simulink model "MX_Gateway"  (Simulink 9.0)
settings: Solver=FixedStepDiscrete  StartTime=0.0  StopTime=100  FixedStep=0.01  RelTol=1e-3
values from neighbouring files (symbols this model uses):
  ACTIVE = Bobcat.Parameter; CreateDefine( ACTIVE                         ,1                          ,'Define' ,'defines.h' )    [defines.m]
  ACTIVE_MODE_ONE = Bobcat.Parameter; CreateDefine( ACTIVE_MODE_ONE                ,19                         ,'Define' ,'defines.h' )    [defines.m]
  AUTHENTICATED = Bobcat.Parameter; CreateDefine( AUTHENTICATED                  ,1                          ,'Define' ,'defines.h' )    [defines.m]
  AUX_EMEA_CONTROLS = Bobcat.Parameter; CreateDefine( AUX_EMEA_CONTROLS  ,1   ,'Define' ,'defines.h' )    [enums.m]
  AUX_FLOW_HIGH = Bobcat.Parameter; CreateDefine( AUX_FLOW_HIGH           ,4  ,'Define' ,'defines.h' )    [enums.m]
  AUX_FLOW_INACTIVE = Bobcat.Parameter; CreateDefine( AUX_FLOW_INACTIVE       ,0  ,'Define' ,'defines.h' )    [enums.m]
  AUX_FLOW_LOW = Bobcat.Parameter; CreateDefine( AUX_FLOW_LOW            ,1  ,'Define' ,'defines.h' )    [enums.m]
  AUX_FLOW_MEDIUM = Bobcat.Parameter; CreateDefine( AUX_FLOW_MEDIUM         ,2  ,'Define' ,'defines.h' )    [enums.m]
  AUX_FLOW_STANDARD = Bobcat.Parameter; CreateDefine( AUX_FLOW_STANDARD       ,3  ,'Define' ,'defines.h' )    [enums.m]
  AUX_NON_FUNCTIONAL = Bobcat.Parameter; CreateDefine( AUX_NON_FUNCTIONAL ,255 ,'Define' ,'defines.h' )    [enums.m]
  BASE_ACTIVE = Bobcat.Parameter; CreateDefine( BASE_ACTIVE                    ,253                        ,'Define' ,'defines.h' )    [defines.m]
  BASE_DETENT = Bobcat.Parameter; CreateDefine( BASE_DETENT                    ,1                          ,'Define' ,'defines.h' )    [defines.m]
  BEEP_NONE = Bobcat.Parameter; CreateDefine( BEEP_NONE                      ,255                        ,'Define' ,'defines.h' )    [defines.m]
  CD_AIR_FILTER_DEBOUNCE_NORMAL = Bobcat.Parameter; CreateAliasCD( CD_AIR_FILTER_DEBOUNCE_NORMAL                        ,uint16(500)                ,'ConstantDataPointer' ,'CONSTANT_DATA' ,'AIR_FILTER_DEBOUNCE_NORMAL')    [cdata.m]    (also in hc12_cdata.m; m4_cdata.m)
  CD_AIR_FILTER_DEBOUNCE_NOT_CONNECTED = Bobcat.Parameter; CreateAliasCD( CD_AIR_FILTER_DEBOUNCE_NOT_CONNECTED                 ,uint16(3000)               ,'ConstantDataPointer' ,'CONSTANT_DATA' ,'AIR_FILTER_DEBOUNCE_NOT_CONNECTED')    [cdata.m]    (also in hc12_cdata.m; m4_cdata.m)
  CD_AIR_FILTER_DEBOUNCE_PLUGGED = Bobcat.Parameter; CreateAliasCD( CD_AIR_FILTER_DEBOUNCE_PLUGGED                       ,uint16(3000)               ,'ConstantDataPointer' ,'CONSTANT_DATA' ,'AIR_FILTER_DEBOUNCE_PLUGGED')    [cdata.m]    (also in hc12_cdata.m; m4_cdata.m)
  CD_AIR_FILTER_FUNCTION = Bobcat.Parameter; CreateAliasCD( CD_AIR_FILTER_FUNCTION                               ,uint8(255)                 ,'ConstantDataPointer' ,'CONSTANT_DATA' ,'AIR_FILTER_FUNCTION')    [cdata.m]    (also in hc12_cdata.m; m4_cdata.m)
  CD_AIR_FILTER_PLUGGED_WEIGHT = Bobcat.Parameter; CreateAliasCD( CD_AIR_FILTER_PLUGGED_WEIGHT                         ,uint8(2)                   ,'ConstantDataPointer' ,'CONSTANT_DATA' ,'AIR_FILTER_PLUGGED_WEIGHT')    [cdata.m]    (also in hc12_cdata.m; m4_cdata.m)
  CD_AIR_FILTER_UNPLUGGED_WEIGHT = Bobcat.Parameter; CreateAliasCD( CD_AIR_FILTER_UNPLUGGED_WEIGHT                       ,uint8(1)                   ,'ConstantDataPointer' ,'CONSTANT_DATA' ,'AIR_FILTER_UNPLUGGED_WEIGHT')    [cdata.m]    (also in hc12_cdata.m; m4_cdata.m)
  CD_AUTOIDLE_ARMBAR_DELAY = Bobcat.Parameter; CreateAliasCD( CD_AUTOIDLE_ARMBAR_DELAY                             ,uint8(2)                   ,'ConstantDataPointer' ,'CONSTANT_DATA' ,'AUTOIDLE_ARMBAR_DELAY')    [cdata.m]    (also in hc12_cdata.m; m4_cdata.m)
  CD_AUTOIDLE_FUNCTION = Bobcat.Parameter; CreateAliasCD( CD_AUTOIDLE_FUNCTION                                 ,uint8(255)                 ,'ConstantDataPointer' ,'CONSTANT_DATA' ,'AUTOIDLE_FUNCTION')    [cdata.m]    (also in hc12_cdata.m; m4_cdata.m)
  CD_AUTOIDLE_IDLE_DELAY = Bobcat.Parameter; CreateAliasCD( CD_AUTOIDLE_IDLE_DELAY                               ,uint8(40)                  ,'ConstantDataPointer' ,'CONSTANT_DATA' ,'AUTOIDLE_IDLE_DELAY')    [cdata.m]    (also in hc12_cdata.m; m4_cdata.m)
  CD_AUTOIDLE_LOW_IDLE_START = Bobcat.Parameter; CreateAliasCD( CD_AUTOIDLE_LOW_IDLE_START                           ,uint8(255)                 ,'ConstantDataPointer' ,'CONSTANT_DATA' ,'AUTOIDLE_LOW_IDLE_START')    [cdata.m]    (also in hc12_cdata.m; m4_cdata.m)
  CD_AUTOIDLE_PRESSURE_OFF = Bobcat.Parameter; CreateAliasCD( CD_AUTOIDLE_PRESSURE_OFF                             ,uint16(300)                ,'ConstantDataPointer' ,'CONSTANT_DATA' ,'AUTOIDLE_PRESSURE_OFF')    [cdata.m]    (also in hc12_cdata.m; m4_cdata.m)
  CD_AUTOIDLE_PRESSURE_ON = Bobcat.Parameter; CreateAliasCD( CD_AUTOIDLE_PRESSURE_ON                              ,uint16(270)                ,'ConstantDataPointer' ,'CONSTANT_DATA' ,'AUTOIDLE_PRESSURE_ON')    [cdata.m]    (also in hc12_cdata.m; m4_cdata.m)
  CD_AUTOSHIFT_FUNCTION = Bobcat.Parameter; CreateAliasCD( CD_AUTOSHIFT_FUNCTION                                ,uint8(255)                 ,'ConstantDataPointer' ,'CONSTANT_DATA' ,'AUTOSHIFT_FUNCTION')    [cdata.m]    (also in hc12_cdata.m; m4_cdata.m)
  CD_AUTOSHIFT_PHIGH1 = Bobcat.Parameter; CreateAliasCD( CD_AUTOSHIFT_PHIGH1                                  ,uint16(205)                ,'ConstantDataPointer' ,'CONSTANT_DATA' ,'AUTOSHIFT_PHIGH1')    [cdata.m]    (also in hc12_cdata.m; m4_cdata.m)
  CD_AUTOSHIFT_PHIGH2 = Bobcat.Parameter; CreateAliasCD( CD_AUTOSHIFT_PHIGH2                                  ,uint16(193)                ,'ConstantDataPointer' ,'CONSTANT_DATA' ,'AUTOSHIFT_PHIGH2')    [cdata.m]    (also in hc12_cdata.m; m4_cdata.m)
  CD_AUTOSHIFT_PHIGH_TEMP1 = Bobcat.Parameter; CreateAliasCD( CD_AUTOSHIFT_PHIGH_TEMP1                             ,int16(70)                  ,'ConstantDataPointer' ,'CONSTANT_DATA' ,'AUTOSHIFT_PHIGH_TEMP1')    [cdata.m]    (also in hc12_cdata.m; m4_cdata.m)
  CD_AUTOSHIFT_PHIGH_TEMP2 = Bobcat.Parameter; CreateAliasCD( CD_AUTOSHIFT_PHIGH_TEMP2                             ,int16(160)                 ,'ConstantDataPointer' ,'CONSTANT_DATA' ,'AUTOSHIFT_PHIGH_TEMP2')    [cdata.m]    (also in hc12_cdata.m; m4_cdata.m)
  CD_AUTOSHIFT_PLOW1 = Bobcat.Parameter; CreateAliasCD( CD_AUTOSHIFT_PLOW1                                   ,uint16(130)                ,'ConstantDataPointer' ,'CONSTANT_DATA' ,'AUTOSHIFT_PLOW1')    [cdata.m]    (also in hc12_cdata.m; m4_cdata.m)
  CD_AUTOSHIFT_PLOW2 = Bobcat.Parameter; CreateAliasCD( CD_AUTOSHIFT_PLOW2                                   ,uint16(100)                ,'ConstantDataPointer' ,'CONSTANT_DATA' ,'AUTOSHIFT_PLOW2')    [cdata.m]    (also in hc12_cdata.m; m4_cdata.m)
  CD_AUTOSHIFT_PLOW_TEMP1 = Bobcat.Parameter; CreateAliasCD( CD_AUTOSHIFT_PLOW_TEMP1                              ,int16(20)                  ,'ConstantDataPointer' ,'CONSTANT_DATA' ,'AUTOSHIFT_PLOW_TEMP1')    [cdata.m]    (also in hc12_cdata.m; m4_cdata.m)
  CD_AUTOSHIFT_PLOW_TEMP2 = Bobcat.Parameter; CreateAliasCD( CD_AUTOSHIFT_PLOW_TEMP2                              ,int16(80)                  ,'ConstantDataPointer' ,'CONSTANT_DATA' ,'AUTOSHIFT_PLOW_TEMP2')    [cdata.m]    (also in hc12_cdata.m; m4_cdata.m)
  CD_AUTOSHIFT_TRAVEL_DELAY = Bobcat.Parameter; CreateAliasCD( CD_AUTOSHIFT_TRAVEL_DELAY                            ,uint8(32)                  ,'ConstantDataPointer' ,'CONSTANT_DATA' ,'AUTOSHIFT_TRAVEL_DELAY')    [cdata.m]    (also in hc12_cdata.m; m4_cdata.m)
  CD_AUX2_CRACKPOINT_CURRENT = Bobcat.Parameter; CreateAliasCD( CD_AUX2_CRACKPOINT_CURRENT                           ,fi(0,0,16,12,'hex','0c28') ,'ConstantDataPointer' ,'CONSTANT_DATA' ,'AUX2_CRACKPOINT_CURRENT')    [cdata.m]    (also in hc12_cdata.m; m4_cdata.m)
  CD_AUX2_FLOW_LOW_FUNCTION = Bobcat.Parameter; CreateAliasCD( CD_AUX2_FLOW_LOW_FUNCTION                            ,uint8(255)                 ,'ConstantDataPointer' ,'CONSTANT_DATA' ,'AUX2_FLOW_LOW_FUNCTION')    [cdata.m]    (also in hc12_cdata.m; m4_cdata.m)
  CD_AUX2_FLOW_MED_FUNCTION = Bobcat.Parameter; CreateAliasCD( CD_AUX2_FLOW_MED_FUNCTION                            ,uint8(255)                 ,'ConstantDataPointer' ,'CONSTANT_DATA' ,'AUX2_FLOW_MED_FUNCTION')    [cdata.m]    (also in hc12_cdata.m; m4_cdata.m)
  CD_AUX2_HI_FLOW_PERCENT = Bobcat.Parameter; CreateAliasCD( CD_AUX2_HI_FLOW_PERCENT                              ,fi(0,0,16,16,'hex','ffff') ,'ConstantDataPointer' ,'CONSTANT_DATA' ,'AUX2_HI_FLOW_PERCENT')    [cdata.m]    (also in hc12_cdata.m; m4_cdata.m)
  CD_AUX2_LOW_FLOW_PERCENT = Bobcat.Parameter; CreateAliasCD( CD_AUX2_LOW_FLOW_PERCENT                             ,fi(0,0,16,16,'hex','7333') ,'ConstantDataPointer' ,'CONSTANT_DATA' ,'AUX2_LOW_FLOW_PERCENT')    [cdata.m]    (also in hc12_cdata.m; m4_cdata.m)
  CD_AUX2_MAX_FLOW_CURRENT = Bobcat.Parameter; CreateAliasCD( CD_AUX2_MAX_FLOW_CURRENT                             ,fi(0,0,16,12,'hex','14cc') ,'ConstantDataPointer' ,'CONSTANT_DATA' ,'AUX2_MAX_FLOW_CURRENT')    [cdata.m]    (also in hc12_cdata.m; m4_cdata.m)
  CD_AUX2_MED_FLOW_PERCENT = Bobcat.Parameter; CreateAliasCD( CD_AUX2_MED_FLOW_PERCENT                             ,fi(0,0,16,16,'hex','9999') ,'ConstantDataPointer' ,'CONSTANT_DATA' ,'AUX2_MED_FLOW_PERCENT')    [cdata.m]    (also in hc12_cdata.m; m4_cdata.m)
  CD_AUX_CRACKPOINT_CURRENT = Bobcat.Parameter; CreateAliasCD( CD_AUX_CRACKPOINT_CURRENT                            ,fi(0,0,16,12,'hex','0000') ,'ConstantDataPointer' ,'CONSTANT_DATA' ,'AUX_CRACKPOINT_CURRENT')    [cdata.m]    (also in hc12_cdata.m; m4_cdata.m)
  CD_AUX_DETENT_FLASH_TIME = Bobcat.Parameter; CreateAliasCD( CD_AUX_DETENT_FLASH_TIME                             ,uint16(72)                 ,'ConstantDataPointer' ,'CONSTANT_DATA' ,'AUX_DETENT_FLASH_TIME')    [cdata.m]    (also in hc12_cdata.m; m4_cdata.m)
  CD_AUX_DETENT_HOLD_TIME = Bobcat.Parameter; CreateAliasCD( CD_AUX_DETENT_HOLD_TIME                              ,uint16(100)                ,'ConstantDataPointer' ,'CONSTANT_DATA' ,'AUX_DETENT_HOLD_TIME')    [cdata.m]    (also in hc12_cdata.m; m4_cdata.m)
  CD_AUX_FULL_ON_AUX1 = Bobcat.Parameter; CreateAliasCD( CD_AUX_FULL_ON_AUX1                                  ,fi(0,0,16,12,'hex','10cd') ,'ConstantDataPointer' ,'CONSTANT_DATA' ,'AUX_FULL_ON_AUX1')    [cdata.m]    (also in hc12_cdata.m; m4_cdata.m)
  CD_AUX_FULL_ON_AUX2 = Bobcat.Parameter; CreateAliasCD( CD_AUX_FULL_ON_AUX2                                  ,fi(0,0,16,12,'hex','139d') ,'ConstantDataPointer' ,'CONSTANT_DATA' ,'AUX_FULL_ON_AUX2')    [cdata.m]    (also in hc12_cdata.m; m4_cdata.m)
  CD_AUX_FULL_ON_AUX3 = Bobcat.Parameter; CreateAliasCD( CD_AUX_FULL_ON_AUX3                                  ,fi(0,0,16,12,'hex','17d7') ,'ConstantDataPointer' ,'CONSTANT_DATA' ,'AUX_FULL_ON_AUX3')    [cdata.m]    (also in hc12_cdata.m; m4_cdata.m)
  CD_AUX_FUNCTION = Bobcat.Parameter; CreateAliasCD( CD_AUX_FUNCTION                                      ,uint8(0)                   ,'ConstantDataPointer' ,'CONSTANT_DATA' ,'AUX_FUNCTION')    [cdata.m]    (also in hc12_cdata.m; m4_cdata.m)
  CD_AUX_RATE_LIMIT_CRACKPOINT = Bobcat.Parameter; CreateAliasCD( CD_AUX_RATE_LIMIT_CRACKPOINT                         ,fi(0,0,16,12,'hex','0800') ,'ConstantDataPointer' ,'CONSTANT_DATA' ,'AUX_RATE_LIMIT_CRACKPOINT')    [cdata.m]    (also in hc12_cdata.m; m4_cdata.m)
  CD_AUX_RATE_LIMIT_CRACKPOINT_RATE = Bobcat.Parameter; CreateAliasCD( CD_AUX_RATE_LIMIT_CRACKPOINT_RATE                    ,fi(0,1,32,12,'int',102400) ,'ConstantDataPointer' ,'CONSTANT_DATA' ,'AUX_RATE_LIMIT_CRACKPOINT_RATE')    [cdata.m]    (also in hc12_cdata.m; m4_cdata.m)
  CD_AUX_RATE_LIMIT_FUNCTION = Bobcat.Parameter; CreateAliasCD( CD_AUX_RATE_LIMIT_FUNCTION                           ,uint8(255)                 ,'ConstantDataPointer' ,'CONSTANT_DATA' ,'AUX_RATE_LIMIT_FUNCTION')    [cdata.m]    (also in hc12_cdata.m; m4_cdata.m)
  CD_AUX_RATE_LIMIT_MAX_ENG_SPD = Bobcat.Parameter; CreateAliasCD( CD_AUX_RATE_LIMIT_MAX_ENG_SPD                        ,uint16(1600)               ,'ConstantDataPointer' ,'CONSTANT_DATA' ,'AUX_RATE_LIMIT_MAX_ENG_SPD')    [cdata.m]    (also in hc12_cdata.m; m4_cdata.m)
  CD_AUX_RATE_LIMIT_MIN_ENG_SPD = Bobcat.Parameter; CreateAliasCD( CD_AUX_RATE_LIMIT_MIN_ENG_SPD                        ,uint16(100)                ,'ConstantDataPointer' ,'CONSTANT_DATA' ,'AUX_RATE_LIMIT_MIN_ENG_SPD')    [cdata.m]    (also in hc12_cdata.m; m4_cdata.m)
  CD_AUX_RATE_LIMIT_NORMAL_RATE = Bobcat.Parameter; CreateAliasCD( CD_AUX_RATE_LIMIT_NORMAL_RATE                        ,fi(0,1,32,12,'int',2048)   ,'ConstantDataPointer' ,'CONSTANT_DATA' ,'AUX_RATE_LIMIT_NORMAL_RATE')    [cdata.m]    (also in hc12_cdata.m; m4_cdata.m)
  CD_AUXPOT_CAL_DEADBAND_HIGH = Bobcat.Parameter; CreateAliasCD( CD_AUXPOT_CAL_DEADBAND_HIGH                          ,uint16(574)                ,'ConstantDataPointer' ,'CONSTANT_DATA' ,'AUXPOT_CAL_DEADBAND_HIGH')    [cdata.m]    (also in hc12_cdata.m; m4_cdata.m)
  CD_AUXPOT_CAL_DEADBAND_LOW = Bobcat.Parameter; CreateAliasCD( CD_AUXPOT_CAL_DEADBAND_LOW                           ,uint16(424)                ,'ConstantDataPointer' ,'CONSTANT_DATA' ,'AUXPOT_CAL_DEADBAND_LOW')    [cdata.m]    (also in hc12_cdata.m; m4_cdata.m)
  CD_AUXPOT_CENTER_RATIO = Bobcat.Parameter; CreateAliasCD( CD_AUXPOT_CENTER_RATIO                               ,uint16(500)                ,'ConstantDataPointer' ,'CONSTANT_DATA' ,'AUXPOT_CENTER_RATIO')    [cdata.m]    (also in hc12_cdata.m; m4_cdata.m)
  CD_AUXPOT_DEADBAND = Bobcat.Parameter; CreateAliasCD( CD_AUXPOT_DEADBAND                                   ,uint8(38)                  ,'ConstantDataPointer' ,'CONSTANT_DATA' ,'AUXPOT_DEADBAND')    [cdata.m]    (also in hc12_cdata.m; m4_cdata.m)
  CD_AUXPOT_FUNCTION = Bobcat.Parameter; CreateAliasCD( CD_AUXPOT_FUNCTION                                   ,uint8(0)                   ,'ConstantDataPointer' ,'CONSTANT_DATA' ,'AUXPOT_FUNCTION')    [cdata.m]    (also in hc12_cdata.m; m4_cdata.m)
  CD_AUXPOT_RATIO_GAIN = Bobcat.Parameter; CreateAliasCD( CD_AUXPOT_RATIO_GAIN                                 ,uint16(1000)               ,'ConstantDataPointer' ,'CONSTANT_DATA' ,'AUXPOT_RATIO_GAIN')    [cdata.m]    (also in hc12_cdata.m; m4_cdata.m)
  CD_CAN_THROTTLE_FUNCTION = Bobcat.Parameter; CreateAliasCD( CD_CAN_THROTTLE_FUNCTION                             ,uint8(255)                 ,'ConstantDataPointer' ,'CONSTANT_DATA' ,'CAN_THROTTLE_FUNCTION')    [cdata.m]    (also in hc12_cdata.m; m4_cdata.m)
  CD_CAN_THROTTLE_REVERSE = Bobcat.Parameter; CreateAliasCD( CD_CAN_THROTTLE_REVERSE                              ,uint8(255)                 ,'ConstantDataPointer' ,'CONSTANT_DATA' ,'CAN_THROTTLE_REVERSE')    [cdata.m]    (also in hc12_cdata.m; m4_cdata.m)
  CD_CONSOLE_DEBOUNCE_ABSENT = Bobcat.Parameter; CreateAliasCD( CD_CONSOLE_DEBOUNCE_ABSENT                           ,uint16(12)                 ,'ConstantDataPointer' ,'CONSTANT_DATA' ,'CONSOLE_DEBOUNCE_ABSENT')    [cdata.m]    (also in hc12_cdata.m; m4_cdata.m)
  CD_CONSOLE_DEBOUNCE_OORH = Bobcat.Parameter; CreateAliasCD( CD_CONSOLE_DEBOUNCE_OORH                             ,uint16(12)                 ,'ConstantDataPointer' ,'CONSTANT_DATA' ,'CONSOLE_DEBOUNCE_OORH')    [cdata.m]    (also in hc12_cdata.m; m4_cdata.m)
  CD_CONSOLE_DEBOUNCE_OORL = Bobcat.Parameter; CreateAliasCD( CD_CONSOLE_DEBOUNCE_OORL                             ,uint16(12)                 ,'ConstantDataPointer' ,'CONSTANT_DATA' ,'CONSOLE_DEBOUNCE_OORL')    [cdata.m]    (also in hc12_cdata.m; m4_cdata.m)
  CD_CONSOLE_DEBOUNCE_PRESENT = Bobcat.Parameter; CreateAliasCD( CD_CONSOLE_DEBOUNCE_PRESENT                          ,uint16(12)                 ,'ConstantDataPointer' ,'CONSTANT_DATA' ,'CONSOLE_DEBOUNCE_PRESENT')    [cdata.m]    (also in hc12_cdata.m; m4_cdata.m)
  CD_CONSOLE_FUNCTION = Bobcat.Parameter; CreateAliasCD( CD_CONSOLE_FUNCTION                                  ,uint8(0)                   ,'ConstantDataPointer' ,'CONSTANT_DATA' ,'CONSOLE_FUNCTION')    [cdata.m]    (also in hc12_cdata.m; m4_cdata.m)
  CD_CONSOLE_OORH = Bobcat.Parameter; CreateAliasCD( CD_CONSOLE_OORH                                      ,uint16(740)                ,'ConstantDataPointer' ,'CONSTANT_DATA' ,'CONSOLE_OORH')    [cdata.m]    (also in hc12_cdata.m; m4_cdata.m)
  CD_CONSOLE_OORL = Bobcat.Parameter; CreateAliasCD( CD_CONSOLE_OORL                                      ,uint16(10)                 ,'ConstantDataPointer' ,'CONSTANT_DATA' ,'CONSOLE_OORL')    [cdata.m]    (also in hc12_cdata.m; m4_cdata.m)
  CD_CONSOLE_PRESENT_HIGH = Bobcat.Parameter; CreateAliasCD( CD_CONSOLE_PRESENT_HIGH                              ,uint16(515)                ,'ConstantDataPointer' ,'CONSTANT_DATA' ,'CONSOLE_PRESENT_HIGH')    [cdata.m]    (also in hc12_cdata.m; m4_cdata.m)
  CD_CONSOLE_PRESENT_LOW = Bobcat.Parameter; CreateAliasCD( CD_CONSOLE_PRESENT_LOW                               ,uint16(375)                ,'ConstantDataPointer' ,'CONSTANT_DATA' ,'CONSOLE_PRESENT_LOW')    [cdata.m]    (also in hc12_cdata.m; m4_cdata.m)
  CD_DELUXE_GEN5_5_FUNCTION = Bobcat.Parameter; CreateAliasCD( CD_DELUXE_GEN5_5_FUNCTION                            ,uint8(255)                 ,'ConstantDataPointer' ,'CONSTANT_DATA' ,'DELUXE_GEN5_5_FUNCTION')    [cdata.m]    (also in hc12_cdata.m; m4_cdata.m)
  CD_DELUXE_GEN5_FUNCTION = Bobcat.Parameter; CreateAliasCD( CD_DELUXE_GEN5_FUNCTION                              ,uint8(255)                 ,'ConstantDataPointer' ,'CONSTANT_DATA' ,'DELUXE_GEN5_FUNCTION')    [cdata.m]    (also in hc12_cdata.m; m4_cdata.m)
  CD_DERATE_ENGINE_SPEED_DEBOUNCE_HIGH = Bobcat.Parameter; CreateAliasCD( CD_DERATE_ENGINE_SPEED_DEBOUNCE_HIGH                 ,uint16(200)                ,'ConstantDataPointer' ,'CONSTANT_DATA' ,'DERATE_ENGINE_SPEED_DEBOUNCE_HIGH')    [cdata.m]    (also in hc12_cdata.m; m4_cdata.m)
  CD_DERATE_ENGINE_SPEED_DEBOUNCE_SHUTDOWN = Bobcat.Parameter; CreateAliasCD( CD_DERATE_ENGINE_SPEED_DEBOUNCE_SHUTDOWN             ,uint16(1000)               ,'ConstantDataPointer' ,'CONSTANT_DATA' ,'DERATE_ENGINE_SPEED_DEBOUNCE_SHUTDOWN')    [cdata.m]    (also in hc12_cdata.m; m4_cdata.m)
  CD_DERATE_ENGINE_SPEED_HIGH_OFFSET = Bobcat.Parameter; CreateAliasCD( CD_DERATE_ENGINE_SPEED_HIGH_OFFSET                   ,uint16(200)                ,'ConstantDataPointer' ,'CONSTANT_DATA' ,'DERATE_ENGINE_SPEED_HIGH_OFFSET')    [cdata.m]    (also in hc12_cdata.m; m4_cdata.m)
  CD_DERATE_METHOD = Bobcat.Parameter; CreateAliasCD( CD_DERATE_METHOD                                     ,uint8(0)                   ,'ConstantDataPointer' ,'CONSTANT_DATA' ,'DERATE_METHOD')    [cdata.m]    (also in hc12_cdata.m; m4_cdata.m)
  CD_DUMP_VALVE_FUNCTION = Bobcat.Parameter; CreateAliasCD( CD_DUMP_VALVE_FUNCTION                               ,uint8(255)                 ,'ConstantDataPointer' ,'CONSTANT_DATA' ,'DUMP_VALVE_FUNCTION')    [cdata.m]    (also in hc12_cdata.m; m4_cdata.m)
  CD_DUMP_VALVE_ON_CURRENT = Bobcat.Parameter; CreateAliasCD( CD_DUMP_VALVE_ON_CURRENT                             ,fi(0,0,16,12,'hex','ffff') ,'ConstantDataPointer' ,'CONSTANT_DATA' ,'DUMP_VALVE_ON_CURRENT')    [cdata.m]    (also in hc12_cdata.m; m4_cdata.m)
  CD_ECO_MODE_THROTTLE_REDUCTION = Bobcat.Parameter; CreateAliasCD( CD_ECO_MODE_THROTTLE_REDUCTION                       ,uint16(850)                ,'ConstantDataPointer' ,'CONSTANT_DATA' ,'ECO_MODE_THROTTLE_REDUCTION')    [cdata.m]    (also in hc12_cdata.m; m4_cdata.m)
  CD_ECT_FILTER_GAIN = Bobcat.Parameter; CreateAliasCD( CD_ECT_FILTER_GAIN                                   ,uint8(6)                   ,'ConstantDataPointer' ,'CONSTANT_DATA' ,'ECT_FILTER_GAIN')    [cdata.m]    (also in hc12_cdata.m; m4_cdata.m)
  CD_ECU_FUNCTION = Bobcat.Parameter; CreateAliasCD( CD_ECU_FUNCTION                                      ,uint8(255)                 ,'ConstantDataPointer' ,'CONSTANT_DATA' ,'ECU_FUNCTION')    [cdata.m]    (also in hc12_cdata.m; m4_cdata.m)
  CD_ECU_HOLD_DEBOUNCE_ERROR_ON = Bobcat.Parameter; CreateAliasCD( CD_ECU_HOLD_DEBOUNCE_ERROR_ON                        ,uint16(20)                 ,'ConstantDataPointer' ,'CONSTANT_DATA' ,'ECU_HOLD_DEBOUNCE_ERROR_ON')    [cdata.m]    (also in hc12_cdata.m; m4_cdata.m)
  CD_EMEA_CONTROLS_HOLD_TIME = Bobcat.Parameter; CreateAliasCD( CD_EMEA_CONTROLS_HOLD_TIME                           ,uint16(30)                 ,'ConstantDataPointer' ,'CONSTANT_DATA' ,'EMEA_CONTROLS_HOLD_TIME')    [cdata.m]    (also in hc12_cdata.m; m4_cdata.m)
  CD_EMEA_CONTROLS_OPTION_A = Bobcat.Parameter; CreateAliasCD( CD_EMEA_CONTROLS_OPTION_A                            ,uint8(255)                 ,'ConstantDataPointer' ,'CONSTANT_DATA' ,'EMEA_CONTROLS_OPTION_A')    [cdata.m]    (also in hc12_cdata.m; m4_cdata.m)
  CD_EMEA_CONTROLS_OPTION_C = Bobcat.Parameter; CreateAliasCD( CD_EMEA_CONTROLS_OPTION_C                            ,uint8(255)                 ,'ConstantDataPointer' ,'CONSTANT_DATA' ,'EMEA_CONTROLS_OPTION_C')    [cdata.m]    (also in hc12_cdata.m; m4_cdata.m)
  CD_EMEA_CONTROLS_REVERSE = Bobcat.Parameter; CreateAliasCD( CD_EMEA_CONTROLS_REVERSE                             ,uint8(255)                 ,'ConstantDataPointer' ,'CONSTANT_DATA' ,'EMEA_CONTROLS_REVERSE')    [cdata.m]    (also in hc12_cdata.m; m4_cdata.m)
  CD_EMEA_ELECTRIC_VERSION = Bobcat.Parameter; CreateAliasCD( CD_EMEA_ELECTRIC_VERSION                             ,uint8(0)                   ,'ConstantDataPointer' ,'CONSTANT_DATA' ,'EMEA_ELECTRIC_VERSION')    [cdata.m]    (also in hc12_cdata.m; m4_cdata.m)
  CD_EMEA_POT_CAL_DEADBAND_HIGH = Bobcat.Parameter; CreateAliasCD( CD_EMEA_POT_CAL_DEADBAND_HIGH                        ,uint16(574)                ,'ConstantDataPointer' ,'CONSTANT_DATA' ,'EMEA_POT_CAL_DEADBAND_HIGH')    [cdata.m]    (also in hc12_cdata.m; m4_cdata.m)
  CD_EMEA_POT_CAL_DEADBAND_LOW = Bobcat.Parameter; CreateAliasCD( CD_EMEA_POT_CAL_DEADBAND_LOW                         ,uint16(424)                ,'ConstantDataPointer' ,'CONSTANT_DATA' ,'EMEA_POT_CAL_DEADBAND_LOW')    [cdata.m]    (also in hc12_cdata.m; m4_cdata.m)
  CD_ENGINE_RPM_FACTOR = Bobcat.Parameter; CreateAliasCD( CD_ENGINE_RPM_FACTOR                                 ,uint8(35)                  ,'ConstantDataPointer' ,'CONSTANT_DATA' ,'ENGINE_RPM_FACTOR')    [cdata.m]    (also in hc12_cdata.m; m4_cdata.m)
  CD_ENGINE_SPEED_FILTER_FAST_VALUE = Bobcat.Parameter; CreateAliasCD( CD_ENGINE_SPEED_FILTER_FAST_VALUE                    ,uint8(1)                   ,'ConstantDataPointer' ,'CONSTANT_DATA' ,'ENGINE_SPEED_FILTER_FAST_VALUE')    [cdata.m]    (also in hc12_cdata.m; m4_cdata.m)
  CD_ENGINE_SPEED_FILTER_SLOW_VALUE = Bobcat.Parameter; CreateAliasCD( CD_ENGINE_SPEED_FILTER_SLOW_VALUE                    ,uint8(4)                   ,'ConstantDataPointer' ,'CONSTANT_DATA' ,'ENGINE_SPEED_FILTER_SLOW_VALUE')    [cdata.m]    (also in hc12_cdata.m; m4_cdata.m)
  CD_ENGINE_SPEED_RUNNING = Bobcat.Parameter; CreateAliasCD( CD_ENGINE_SPEED_RUNNING                              ,uint16(800)                ,'ConstantDataPointer' ,'CONSTANT_DATA' ,'ENGINE_SPEED_RUNNING')    [cdata.m]    (also in hc12_cdata.m; m4_cdata.m)
  CD_ENGINE_START_DELAY_DEBOUNCE_RPM_NO_SIGNAL = Bobcat.Parameter; CreateAliasCD( CD_ENGINE_START_DELAY_DEBOUNCE_RPM_NO_SIGNAL         ,uint16(0)                  ,'ConstantDataPointer' ,'CONSTANT_DATA' ,'ENGINE_START_DELAY_DEBOUNCE_RPM_NO_SIGNAL')    [cdata.m]    (also in hc12_cdata.m; m4_cdata.m)
  CD_ENGINE_START_DELAY_OFF_TIME = Bobcat.Parameter; CreateAliasCD( CD_ENGINE_START_DELAY_OFF_TIME                       ,uint16(0)                  ,'ConstantDataPointer' ,'CONSTANT_DATA' ,'ENGINE_START_DELAY_OFF_TIME')    [cdata.m]    (also in hc12_cdata.m; m4_cdata.m)
  CD_ENGINE_START_DELAY_RPM_NO_SIGNAL = Bobcat.Parameter; CreateAliasCD( CD_ENGINE_START_DELAY_RPM_NO_SIGNAL                  ,uint16(0)                  ,'ConstantDataPointer' ,'CONSTANT_DATA' ,'ENGINE_START_DELAY_RPM_NO_SIGNAL')    [cdata.m]    (also in hc12_cdata.m; m4_cdata.m)
  CD_EOP_DEBOUNCE_EXTREMELY_LOW = Bobcat.Parameter; CreateAliasCD( CD_EOP_DEBOUNCE_EXTREMELY_LOW                        ,uint16(50)                 ,'ConstantDataPointer' ,'CONSTANT_DATA' ,'EOP_DEBOUNCE_EXTREMELY_LOW')    [cdata.m]    (also in hc12_cdata.m; m4_cdata.m)
  CD_EOP_DEBOUNCE_NORMAL = Bobcat.Parameter; CreateAliasCD( CD_EOP_DEBOUNCE_NORMAL                               ,uint16(50)                 ,'ConstantDataPointer' ,'CONSTANT_DATA' ,'EOP_DEBOUNCE_NORMAL')    [cdata.m]    (also in hc12_cdata.m; m4_cdata.m)
  CD_EOP_DEBOUNCE_SHUTDOWN = Bobcat.Parameter; CreateAliasCD( CD_EOP_DEBOUNCE_SHUTDOWN                             ,uint16(100)                ,'ConstantDataPointer' ,'CONSTANT_DATA' ,'EOP_DEBOUNCE_SHUTDOWN')    [cdata.m]    (also in hc12_cdata.m; m4_cdata.m)
  CD_EOP_FILTER_VALUE = Bobcat.Parameter; CreateAliasCD( CD_EOP_FILTER_VALUE                                  ,uint8(3)                   ,'ConstantDataPointer' ,'CONSTANT_DATA' ,'EOP_FILTER_VALUE')    [cdata.m]    (also in hc12_cdata.m; m4_cdata.m)
  CD_EOP_FUNCTION = Bobcat.Parameter; CreateAliasCD( CD_EOP_FUNCTION                                      ,uint8(1)                   ,'ConstantDataPointer' ,'CONSTANT_DATA' ,'EOP_FUNCTION')    [cdata.m]    (also in hc12_cdata.m; m4_cdata.m)
  CD_EOP_SWITCH_GAUGE_VALUE = Bobcat.Parameter; CreateAliasCD( CD_EOP_SWITCH_GAUGE_VALUE                            ,uint16(125)                ,'ConstantDataPointer' ,'CONSTANT_DATA' ,'EOP_SWITCH_GAUGE_VALUE')    [cdata.m]    (also in hc12_cdata.m; m4_cdata.m)
  CD_EOP_SWITCH_LOW = Bobcat.Parameter; CreateAliasCD( CD_EOP_SWITCH_LOW                                    ,uint16(50)                 ,'ConstantDataPointer' ,'CONSTANT_DATA' ,'EOP_SWITCH_LOW')    [cdata.m]    (also in hc12_cdata.m; m4_cdata.m)
  CD_EOP_SWITCH_PRESSURE_VALUE = Bobcat.Parameter; CreateAliasCD( CD_EOP_SWITCH_PRESSURE_VALUE                         ,uint16(40)                 ,'ConstantDataPointer' ,'CONSTANT_DATA' ,'EOP_SWITCH_PRESSURE_VALUE')    [cdata.m]    (also in hc12_cdata.m; m4_cdata.m)
  CD_EOT_DEBOUNCE_EXTREMELY_LOW = Bobcat.Parameter; CreateAliasCD( CD_EOT_DEBOUNCE_EXTREMELY_LOW                        ,uint16(2)                  ,'ConstantDataPointer' ,'CONSTANT_DATA' ,'EOT_DEBOUNCE_EXTREMELY_LOW')    [cdata.m]    (also in hc12_cdata.m; m4_cdata.m)
  CD_EOT_DEBOUNCE_LOW = Bobcat.Parameter; CreateAliasCD( CD_EOT_DEBOUNCE_LOW                                  ,uint16(2)                  ,'ConstantDataPointer' ,'CONSTANT_DATA' ,'EOT_DEBOUNCE_LOW')    [cdata.m]    (also in hc12_cdata.m; m4_cdata.m)
  CD_EOT_DEBOUNCE_NORMAL = Bobcat.Parameter; CreateAliasCD( CD_EOT_DEBOUNCE_NORMAL                               ,uint16(2)                  ,'ConstantDataPointer' ,'CONSTANT_DATA' ,'EOT_DEBOUNCE_NORMAL')    [cdata.m]    (also in hc12_cdata.m; m4_cdata.m)
  CD_EOT_EXTREMELY_LOW = Bobcat.Parameter; CreateAliasCD( CD_EOT_EXTREMELY_LOW                                 ,uint16(81)                 ,'ConstantDataPointer' ,'CONSTANT_DATA' ,'EOT_EXTREMELY_LOW')    [cdata.m]    (also in hc12_cdata.m; m4_cdata.m)
  CD_EOT_FUNCTION = Bobcat.Parameter; CreateAliasCD( CD_EOT_FUNCTION                                      ,uint8(255)                 ,'ConstantDataPointer' ,'CONSTANT_DATA' ,'EOT_FUNCTION')    [cdata.m]    (also in hc12_cdata.m; m4_cdata.m)
  CD_EOT_LOW = Bobcat.Parameter; CreateAliasCD( CD_EOT_LOW                                           ,uint16(90)                 ,'ConstantDataPointer' ,'CONSTANT_DATA' ,'EOT_LOW')    [cdata.m]    (also in hc12_cdata.m; m4_cdata.m)
  CD_EOT_TEMPERATURE_HYSTERESIS = Bobcat.Parameter; CreateAliasCD( CD_EOT_TEMPERATURE_HYSTERESIS                        ,uint16(2)                  ,'ConstantDataPointer' ,'CONSTANT_DATA' ,'EOT_TEMPERATURE_HYSTERESIS')    [cdata.m]    (also in hc12_cdata.m; m4_cdata.m)
  CD_FAST_TRAVEL_FUNCTION = Bobcat.Parameter; CreateAliasCD( CD_FAST_TRAVEL_FUNCTION                              ,uint8(255)                 ,'ConstantDataPointer' ,'CONSTANT_DATA' ,'FAST_TRAVEL_FUNCTION')    [cdata.m]    (also in hc12_cdata.m; m4_cdata.m)
  CD_FAST_TRAVEL_THROTTLE_DELAY = Bobcat.Parameter; CreateAliasCD( CD_FAST_TRAVEL_THROTTLE_DELAY                        ,uint16(10)                 ,'ConstantDataPointer' ,'CONSTANT_DATA' ,'FAST_TRAVEL_THROTTLE_DELAY')    [cdata.m]    (also in hc12_cdata.m; m4_cdata.m)
  CD_FAST_TRAVEL_THROTTLE_LIMIT = Bobcat.Parameter; CreateAliasCD( CD_FAST_TRAVEL_THROTTLE_LIMIT                        ,uint16(1000)               ,'ConstantDataPointer' ,'CONSTANT_DATA' ,'FAST_TRAVEL_THROTTLE_LIMIT')    [cdata.m]    (also in hc12_cdata.m; m4_cdata.m)
  CD_FLYWHEEL_TEETH = Bobcat.Parameter; CreateAliasCD( CD_FLYWHEEL_TEETH                                    ,uint8(83)                  ,'ConstantDataPointer' ,'CONSTANT_DATA' ,'FLYWHEEL_TEETH')    [cdata.m]    (also in hc12_cdata.m; m4_cdata.m)
  CD_FUEL_LEVEL_FILTER_FAST_VALUE = Bobcat.Parameter; CreateAliasCD( CD_FUEL_LEVEL_FILTER_FAST_VALUE                      ,uint8(5)                   ,'ConstantDataPointer' ,'CONSTANT_DATA' ,'FUEL_LEVEL_FILTER_FAST_VALUE')    [cdata.m]    (also in hc12_cdata.m; m4_cdata.m)
  CD_FUEL_LEVEL_FILTER_SLOW_VALUE = Bobcat.Parameter; CreateAliasCD( CD_FUEL_LEVEL_FILTER_SLOW_VALUE                      ,uint8(10)                  ,'ConstantDataPointer' ,'CONSTANT_DATA' ,'FUEL_LEVEL_FILTER_SLOW_VALUE')    [cdata.m]    (also in hc12_cdata.m; m4_cdata.m)
  CD_FUEL_PRIMARY_FEEDBACK_FUNCTION = Bobcat.Parameter; CreateAliasCD( CD_FUEL_PRIMARY_FEEDBACK_FUNCTION                    ,uint8(0)                   ,'ConstantDataPointer' ,'CONSTANT_DATA' ,'FUEL_PRIMARY_FEEDBACK_FUNCTION')    [cdata.m]    (also in hc12_cdata.m; m4_cdata.m)
  ... (+246 more)
undefined symbols (not defined in the file or in its folder): sigbuilder_block
undefined symbols (not defined in the file): CD_FUEL_RESOLUTION_TIMER
libraries: simulink x186, MX_Library x107
(part 35 of 48)

== stateflow chart MX_Gtwy_Control/PanelInterface/mod_TwoSpeedIcon/RabbitIcon ==
  settings: executeAtInitialization=1
  state INACTIVE
    en:icon_Rabbit = INACTIVE;
  state ERROR
    en:icon_Rabbit = ERROR_FLASH;
  state ACTIVE
    en:icon_Rabbit = ACTIVE;
  (default) -> INACTIVE
  INACTIVE -> ERROR [order 1] : [state_TwoSpeed == SHORT_TO_GROUND...
      | || state_TwoSpeed == SHORT_TO_BATTERY...
      | || state_TwoSpeed == OPEN_CIRCUIT]
  ERROR -> INACTIVE : [state_TwoSpeed != SHORT_TO_GROUND...
      | && state_TwoSpeed != SHORT_TO_BATTERY...
      | && state_TwoSpeed != OPEN_CIRCUIT]
  ACTIVE -> INACTIVE [order 1] : [(state_TwoSpeedIcon == INACTIVE)]
  INACTIVE -> ACTIVE [order 2] : [(state_TwoSpeedIcon == ACTIVE)]
  ACTIVE -> ERROR [order 2] : [state_TwoSpeed == SHORT_TO_GROUND...
      | || state_TwoSpeed == SHORT_TO_BATTERY...
      | || state_TwoSpeed == OPEN_CIRCUIT]
  data: state_TwoSpeed input uint8; icon_Rabbit output uint8; ACTIVE parameter uint8; INACTIVE parameter uint8; SHORT_TO_BATTERY parameter uint8; ERROR_FLASH parameter uint8; state_TwoSpeedIcon input uint8; SHORT_TO_GROUND parameter uint8; OPEN_CIRCUIT parameter uint8

== stateflow chart MX_Gtwy_Control/Outputs/SwitchedPower/Relay ==
  state INACTIVE
    en:dout_SwitchedPwr = INACTIVE;
  state DEBOUNCE_ERROR_ON_CHECK
    en:debounceCount = 0;
    du:debounceCount++;
  state DELAY_OFF
    en:debounceCount = 0;
    du:debounceCount++;
  state ACTIVE
    en:dout_SwitchedPwr = ACTIVE;
  note: Delay switched power relay for a short period of time
      | to allow error detection.  Do not delay if turned on with
      | service tool.  Suitcase service tool turns on switched power
      | which causes a false alarm.
  note: Delay shutdown to give enough time for
      | shutdown message to be sent out so
      | you don't get nuicanse joystick alarms.
  (default) -> INACTIVE
  INACTIVE -> DEBOUNCE_ERROR_ON_CHECK [order 1] : [(state_Key != INACTIVE)...
      | && (din_RemoteKey == INACTIVE)...
      | && (state_System != SYS_POWER_DOWN)...
      | && (state_System != SYS_LIMITED)]
  DELAY_OFF -> INACTIVE : [(debounceCount >= CD_SWITCHED_POWER_RELAY_DEBOUNCE_SHUTDOWN)]
  INACTIVE -> ACTIVE [order 2] : [(din_RemoteKey == ACTIVE)...
      | && (state_System != SYS_POWER_DOWN)]
  DEBOUNCE_ERROR_ON_CHECK -> (junction 2944)
  ACTIVE -> DELAY_OFF : [(state_System == SYS_POWER_DOWN)...
      | || ((state_System == SYS_LIMITED) && (state_Key == INACTIVE))]
  (junction 2944) -> ACTIVE [order 1] : [CD_SWITCHED_POWER_RELAY_DEBOUNCE_ERROR_ON == UNLIMITED]
  (junction 2944) -> (junction 2945) [order 2]
  (junction 2945) -> ACTIVE : [(debounceCount >= (CD_SWITCHED_POWER_RELAY_DEBOUNCE_ERROR_ON + 2))]
  data: state_Key input uint8; dout_SwitchedPwr output boolean; INACTIVE parameter uint8; debounceCount local uint16; CD_SWITCHED_POWER_RELAY_DEBOUNCE_ERROR_ON parameter uint16; din_RemoteKey input uint8; CD_SWITCHED_POWER_RELAY_DEBOUNCE_SHUTDOWN parameter uint16; ACTIVE parameter uint8; state_System input uint8; SYS_POWER_DOWN parameter uint8; SYS_LIMITED parameter uint8; UNLIMITED parameter uint16

== stateflow chart MX_Gtwy_Control/System/ElectricAutomation/Chart2 ==
  state prova
    du:testOut = 0;
  state prova2
    du:testOut = 6;
  state prova1
    du:testOut = 2;
  (default) -> prova
  prova2 -> prova [order 2] : [tempVar == 6]{testOut = 7}
  prova -> prova2 [order 1] : [tempVar == 2]{testOut = 8}
  prova -> prova1 [order 2] : [tempVar == 1]{testOut = 1}
  prova1 -> prova [order 2] : [tempVar == 5]{testOut = 3}
  prova1 -> prova2 [order 1] : [tempVar == 4]{testOut = 4}
  prova2 -> prova1 [order 1] : [tempVar == 3]{testOut = 5}
  data: testOut output double; tempVar input uint8

== stateflow chart MX_Gtwy_Control/Inputs/Load_Moment/Load_Moment_Sensor/Load_Moment ==
  state Invalid
    en:
    state_LoadMoment = NORMAL;
  state Valid
    state Normal
      state Normal
        state Normal
          en:
          state_LoadMoment = NORMAL;
        state Normal_DB
        state Error_DB
        state InError
          en:
          state_LoadMoment = IN_ERROR;
          du:
          state_Buzzer = CONTINUOUS_BEEP;
        (default) -> Valid.Normal.Normal.Normal
        Valid.Normal.Normal.Normal -> Error_DB : [bar_LoadMoment > CD_LOAD_MOMENT_ON]
        Error_DB -> Valid.Normal.Normal.Normal [order 1] : [bar_LoadMoment < CD_LOAD_MOMENT_OFF]
        Normal_DB -> Valid.Normal.Normal.Normal [order 1] : [after(CD_LOAD_MOMENT_NORMAL_DB,tick)]
            | {state_Buzzer = NO_BEEP;}
        InError -> Normal_DB : [bar_LoadMoment < CD_LOAD_MOMENT_OFF]
        Normal_DB -> InError [order 2] : [bar_LoadMoment > CD_LOAD_MOMENT_ON]
        Error_DB -> InError [order 2] : [after(CD_LOAD_MOMENT_ERROR_DB,tick)]
      state Inhibit
        en:
        state_LoadMoment = NORMAL;
      (default) -> Valid.Normal.Normal
      Valid.Normal.Normal -> Inhibit : [din_OWDEnableSw != ACTIVE]
      Inhibit -> Valid.Normal.Normal [order 1] : [din_OWDEnableSw == ACTIVE]
      Inhibit -> Inhibit [order 2] : [state_Buzzer == CONTINUOUS_BEEP]
          | {state_Buzzer = NO_BEEP;}
    state OORL_DB
    state OORL
      entry: state_LoadMoment = OORL;
    state OORL_NORMAL1
    state OORH
      entry: state_LoadMoment = OORH;
    state OORH_DB
    state OORL_NORMAL2
    (default) -> Valid.Normal
    Valid.Normal -> OORL_DB [order 1] : [volts_LoadMoment < CD_LOAD_MOMENT_OORL]
    OORL_DB -> OORL [order 1] : [after(CD_LOAD_MOMENT_DEBOUNCE_OORL ,tick)]
    OORL_DB -> Valid.Normal [order 2] : [volts_LoadMoment >= CD_LOAD_MOMENT_OORL]
    OORL -> OORL_NORMAL1 [order 1] : [volts_LoadMoment >= CD_LOAD_MOMENT_OORL...
        | && CD_LOAD_MOMENT_DEBOUNCE_NORMAL != UNLIMITED ]
    OORL_NORMAL1 -> Valid.Normal [order 1] : [after(CD_LOAD_MOMENT_DEBOUNCE_NORMAL ,tick)]
    OORL_NORMAL1 -> OORL [order 2] : [volts_LoadMoment < CD_LOAD_MOMENT_OORL]
    OORL -> OORL [order 2] : [state_Buzzer == CONTINUOUS_BEEP]
        | {state_Buzzer = NO_BEEP;}
    Valid.Normal -> OORH_DB [order 2] : [volts_LoadMoment > CD_LOAD_MOMENT_OORH]
    OORH_DB -> OORH [order 1] : [after(CD_LOAD_MOMENT_DEBOUNCE_OORH ,tick)]
    OORH_DB -> Valid.Normal [order 2] : [volts_LoadMoment <= CD_LOAD_MOMENT_OORH]
    OORH -> OORL_NORMAL2 [order 1] : [volts_LoadMoment <= CD_LOAD_MOMENT_OORH...
        | && CD_LOAD_MOMENT_DEBOUNCE_NORMAL != UNLIMITED]
    OORL_NORMAL2 -> Valid.Normal [order 2] : [after(CD_LOAD_MOMENT_DEBOUNCE_NORMAL ,tick)]
    OORL_NORMAL2 -> OORH [order 1] : [volts_LoadMoment > CD_LOAD_MOMENT_OORH]
    OORH -> OORH [order 2] : [state_Buzzer == CONTINUOUS_BEEP]
        | {state_Buzzer = NO_BEEP;}
  (default) -> Invalid
  Invalid -> Invalid [order 2] : [state_Buzzer == CONTINUOUS_BEEP]
      | {state_Buzzer = NO_BEEP;}
  Invalid -> Valid [order 1] : [state_SensorSupply == NORMAL]
  Valid -> Invalid : [state_SensorSupply != NORMAL]
  data: NORMAL parameter uint8; CD_LOAD_MOMENT_ERROR_DB parameter uint8; CD_LOAD_MOMENT_NORMAL_DB parameter uint8; IN_ERROR parameter uint8; bar_LoadMoment input uint16; state_LoadMoment output uint8; CD_LOAD_MOMENT_ON parameter uint16; CD_LOAD_MOMENT_OFF parameter uint16; state_Buzzer data_store_memory double; NO_BEEP parameter uint8; CONTINUOUS_BEEP parameter uint8; volts_LoadMoment input uint8; state_SensorSupply input uint8; CD_LOAD_MOMENT_OORL parameter uint8; CD_LOAD_MOMENT_OORH parameter uint8; OORL parameter uint8; OORH parameter uint8; CD_LOAD_MOMENT_DEBOUNCE_OORL parameter uint16; CD_LOAD_MOMENT_DEBOUNCE_OORH parameter uint16; CD_LOAD_MOMENT_DEBOUNCE_NORMAL parameter uint16; UNLIMITED parameter uint16; din_OWDEnableSw input boolean; ACTIVE parameter uint8

== stateflow chart MX_Gtwy_Control/Inputs/EOP/SensorInput/ErrorDetection ==
  state NON_FUNCTIONAL
    state NON_FUNCTIONAL
      en:state_EOPSr = NORMAL;
      en:flt_EOP = 0;
    state ECU_FUNCTION
      en:state_EOPSr = NORMAL;
      du: flt_EOP = ecu_EOP;
    (default) -> NON_FUNCTIONAL.NON_FUNCTIONAL
    NON_FUNCTIONAL.NON_FUNCTIONAL -> ECU_FUNCTION : [(CD_ECU_FUNCTION != NON_FUNCTIONAL)]
  state ECU
    state COMMUNICATING
      du: flt_EOP = ecu_EOP;
      state NORMAL
        state DEBOUNCE_NORMAL
          en:debounceCount = 0;
          du:debounceCount++;
        state NORMAL
          en:state_EOPSr = NORMAL;
        (default) -> DEBOUNCE_NORMAL
        DEBOUNCE_NORMAL -> ECU.COMMUNICATING.NORMAL.NORMAL : [(debounceCount >= CD_EOP_DEBOUNCE_NORMAL)...
            | || (state_EOPSr == NORMAL)]
      state EXTREMELY_LOW
        state DEBOUNCE_EXTREMELY_LOW
          en:debounceCount = 0;
          du:debounceCount++;
        state EXTREMELY_LOW
          en:state_EOPSr = EXTREMELY_LOW;
        (default) -> DEBOUNCE_EXTREMELY_LOW
        DEBOUNCE_EXTREMELY_LOW -> ECU.COMMUNICATING.EXTREMELY_LOW.EXTREMELY_LOW : [(debounceCount >= CD_EOP_DEBOUNCE_EXTREMELY_LOW)...
            | || (state_EOPSr == EXTREMELY_LOW)]
      state SHUTDOWN
        state SHUTDOWN
          en:state_EOPSr = SHUTDOWN;
        state DEBOUNCE
          en:debounceCount = 0;
          du:debounceCount++;
        (default) -> ECU.COMMUNICATING.SHUTDOWN.DEBOUNCE
        ECU.COMMUNICATING.SHUTDOWN.DEBOUNCE -> ECU.COMMUNICATING.SHUTDOWN.SHUTDOWN : [(debounceCount >= CD_EOP_DEBOUNCE_SHUTDOWN)]
      ECU.COMMUNICATING.EXTREMELY_LOW -> ECU.COMMUNICATING.NORMAL [order 1] : [(flt_EOP >= CD_EOP_SWITCH_LOW)...
          | || (state_Engine != ENGINE_RUNNING)]
      ECU.COMMUNICATING.NORMAL -> ECU.COMMUNICATING.EXTREMELY_LOW : [(flt_EOP <= CD_EOP_SWITCH_LOW)...
          | && (state_Engine == ENGINE_RUNNING)]
      ECU.COMMUNICATING.EXTREMELY_LOW -> ECU.COMMUNICATING.SHUTDOWN [order 2] : [(CD_SHUTDOWN_FUNCTION != NON_FUNCTIONAL)...
          | && (state_EOPSr == EXTREMELY_LOW)]
      ECU.COMMUNICATING.SHUTDOWN -> ECU.COMMUNICATING.EXTREMELY_LOW : [((flt_EOP >= CD_EOP_SWITCH_LOW) || (state_Engine != ENGINE_RUNNING))...
          | && (state_EOPSr != SHUTDOWN)]
      (default) -> ECU.COMMUNICATING.NORMAL.NORMAL
    state NO_COMMUNICATION
      en:flt_EOP = 0;
      en:state_EOPSr = NORMAL;
    (default) -> COMMUNICATING
    NO_COMMUNICATION -> COMMUNICATING : [(state_CommECU != NO_COMMUNICATION)]
    COMMUNICATING -> NO_COMMUNICATION : [(state_CommECU == NO_COMMUNICATION)]
  state SENSOR
    state VALUE [parallel]
      settings: executionOrder=1
      state INIT
        en:flt_EOP = 0;
        (default) -> (junction 3101)
        (junction 3101) -> (junction 3102) [order 1] : [((rm_EOP <= CD_EOP_OORL) || (rm_EOP >= CD_EOP_OORH))]
        (junction 3101) -> (junction 3103) [order 2] : {
            | flt_EOP = flt_EOP_in;
            | }
        (junction 3102) -> (junction 3104) : {
            | flt_EOP = 0;
            | }
        (junction 3104) -> (junction 3103)
      state NORMAL
        du:flt_EOP = flt_EOP_in;
      (default) -> INIT
      INIT -> SENSOR.VALUE.NORMAL : [(state_System == SYS_NORMAL)]
    state STATE [parallel]
      settings: executionOrder=2
      state NON_FUNCTIONAL
        en:state_EOPSr = NORMAL;
      state NORMAL
        state NORMAL
          state NORMAL
            entry: state_EOPSr = NORMAL;
          state DEBOUNCE
            en:debounceCount = 0;
            du:debounceCount++;
          (default) -> SENSOR.STATE.NORMAL.NORMAL.DEBOUNCE
          SENSOR.STATE.NORMAL.NORMAL.DEBOUNCE -> SENSOR.STATE.NORMAL.NORMAL.NORMAL : [(debounceCount >= CD_EOP_DEBOUNCE_NORMAL)...
              | || (state_EOPSr == NORMAL)]
        state EXTREMELY_LOW
          state EXTREMELY_LOW
            entry: state_EOPSr = EXTREMELY_LOW;
          state DEBOUNCE
            en:debounceCount = 0;
            du:debounceCount++;
          (default) -> SENSOR.STATE.NORMAL.EXTREMELY_LOW.DEBOUNCE
          SENSOR.STATE.NORMAL.EXTREMELY_LOW.DEBOUNCE -> SENSOR.STATE.NORMAL.EXTREMELY_LOW.EXTREMELY_LOW : [(debounceCount >= CD_EOP_DEBOUNCE_EXTREMELY_LOW)...
              | || (state_EOPSr == EXTREMELY_LOW)]
        state LOW
          state LOW
            entry: state_EOPSr = LOW;
          state DEBOUNCE
            en:debounceCount = 0;
            du:debounceCount++;
          (default) -> SENSOR.STATE.NORMAL.LOW.DEBOUNCE
          SENSOR.STATE.NORMAL.LOW.DEBOUNCE -> SENSOR.STATE.NORMAL.LOW.LOW : [(debounceCount >= CD_EOP_DEBOUNCE_LOW)...
              | || (state_EOPSr == LOW)]
        state SHUTDOWN
          state SHUTDOWN
            entry: state_EOPSr = SHUTDOWN;
          state DEBOUNCE
            en:debounceCount = 0;
            du:debounceCount++;
          (default) -> SENSOR.STATE.NORMAL.SHUTDOWN.DEBOUNCE
          SENSOR.STATE.NORMAL.SHUTDOWN.DEBOUNCE -> SENSOR.STATE.NORMAL.SHUTDOWN.SHUTDOWN : [(debounceCount >= CD_EOP_DEBOUNCE_SHUTDOWN)]
        (default) -> SENSOR.STATE.NORMAL.NORMAL
        SENSOR.STATE.NORMAL.NORMAL -> SENSOR.STATE.NORMAL.EXTREMELY_LOW [order 2] : [(flt_EOP_in <= level_ShutdownEOP)...
            | && (state_Engine == ENGINE_RUNNING)...
            | && (CD_EOP_FAULT_DETECTION_FUNCTION != NON_FUNCTIONAL)]
        SENSOR.STATE.NORMAL.EXTREMELY_LOW -> SENSOR.STATE.NORMAL.NORMAL [order 3] : [(flt_EOP_in > (level_WarningEOP + CD_PRESSURE_HYSTERESIS))...
            | || (state_Engine != ENGINE_RUNNING)]
        SENSOR.STATE.NORMAL.LOW -> SENSOR.STATE.NORMAL.NORMAL [order 1] : [(flt_EOP_in > (level_WarningEOP + CD_PRESSURE_HYSTERESIS))...
            | || (state_Engine != ENGINE_RUNNING)]
        SENSOR.STATE.NORMAL.NORMAL -> SENSOR.STATE.NORMAL.LOW [order 1] : [(flt_EOP_in <= level_WarningEOP)...
            | && (flt_EOP_in > level_ShutdownEOP) ...
            | && (state_Engine == ENGINE_RUNNING)...
            | && (CD_EOP_FAULT_DETECTION_FUNCTION != NON_FUNCTIONAL)]
        SENSOR.STATE.NORMAL.LOW -> SENSOR.STATE.NORMAL.EXTREMELY_LOW [order 2] : [(flt_EOP_in <= level_ShutdownEOP)]
        SENSOR.STATE.NORMAL.EXTREMELY_LOW -> SENSOR.STATE.NORMAL.LOW [order 1] : [(flt_EOP_in > (level_ShutdownEOP + CD_PRESSURE_HYSTERESIS))...
            | && (flt_EOP_in <= level_WarningEOP)]
        SENSOR.STATE.NORMAL.SHUTDOWN -> SENSOR.STATE.NORMAL.LOW : [(flt_EOP_in > (level_ShutdownEOP + CD_PRESSURE_HYSTERESIS))...
            | && (state_EOPSr != SHUTDOWN)]
        SENSOR.STATE.NORMAL.EXTREMELY_LOW -> SENSOR.STATE.NORMAL.SHUTDOWN [order 2] : [(CD_SHUTDOWN_FUNCTION != NON_FUNCTIONAL)...
            | && (state_EOPSr == EXTREMELY_LOW)]
      state OUT_OF_RANGE_HIGH
        state OORH
          entry: state_EOPSr = OORH;
        state DEBOUNCE
          en:debounceCount = 0;
          du:debounceCount++;
        (default) -> SENSOR.STATE.OUT_OF_RANGE_HIGH.DEBOUNCE
        SENSOR.STATE.OUT_OF_RANGE_HIGH.DEBOUNCE -> OORH : [(debounceCount >= CD_EOP_DEBOUNCE_OORH)...
            | || (state_EOPSr == OORH)]
      state OUT_OF_RANGE_LOW
        state OORL
          entry: state_EOPSr = OORL;
        state DEBOUNCE
          en:debounceCount = 0;
          du:debounceCount++;
        (default) -> SENSOR.STATE.OUT_OF_RANGE_LOW.DEBOUNCE
        SENSOR.STATE.OUT_OF_RANGE_LOW.DEBOUNCE -> OORL : [(debounceCount >= CD_EOP_DEBOUNCE_OORL)...
            | || (state_EOPSr == OORL)]
      (default) -> SENSOR.STATE.NON_FUNCTIONAL
      OUT_OF_RANGE_LOW -> SENSOR.STATE.NON_FUNCTIONAL [order 2] : [(state_SensorSupply != NORMAL)...
          | && (state_EOPSr != OORL)]
      OUT_OF_RANGE_HIGH -> SENSOR.STATE.NON_FUNCTIONAL [order 2] : [(state_SensorSupply != NORMAL)...
          | && (state_EOPSr != OORH)]
      SENSOR.STATE.NORMAL -> SENSOR.STATE.NON_FUNCTIONAL [order 1] : [(state_SensorSupply != NORMAL)...
          | || (state_System != SYS_NORMAL)]
      SENSOR.STATE.NON_FUNCTIONAL -> SENSOR.STATE.NORMAL : [(state_SensorSupply == NORMAL)...
          | && (state_System == SYS_NORMAL)]
      SENSOR.STATE.NORMAL -> OUT_OF_RANGE_HIGH [order 3] : [(rm_EOP >= CD_EOP_OORH)...
          | && (state_EOPSr != SHUTDOWN)]
      OUT_OF_RANGE_HIGH -> SENSOR.STATE.NORMAL [order 1] : [(rm_EOP < CD_EOP_OORH)]
      SENSOR.STATE.NORMAL -> OUT_OF_RANGE_LOW [order 2] : [(rm_EOP <= CD_EOP_OORL)...
          | && (state_EOPSr != SHUTDOWN)]
      OUT_OF_RANGE_LOW -> SENSOR.STATE.NORMAL [order 1] : [(rm_EOP > CD_EOP_OORL)]
  (default) -> NON_FUNCTIONAL
  NON_FUNCTIONAL -> ECU [order 2] : [(CD_EOP_FUNCTION != NON_FUNCTIONAL)...
      | && (CD_ECU_FUNCTION != NON_FUNCTIONAL)]
  NON_FUNCTIONAL -> SENSOR [order 1] : [(CD_EOP_FUNCTION != NON_FUNCTIONAL)...
      | && (CD_ECU_FUNCTION == NON_FUNCTIONAL)]
  data: rm_EOP input uint16; flt_EOP_in input uint16; state_EOPSr output uint8; state_SensorSupply input uint8; state_Engine input uint8; CD_EOP_FUNCTION parameter uint8; CD_EOP_OORH parameter uint16; CD_EOP_OORL parameter uint16; CD_EOP_DEBOUNCE_OORH parameter uint16; CD_EOP_DEBOUNCE_OORL parameter uint16; CD_EOP_DEBOUNCE_NORMAL parameter uint16; CD_EOP_DEBOUNCE_LOW parameter uint16; CD_EOP_DEBOUNCE_EXTREMELY_LOW parameter uint16; CD_EOP_DEBOUNCE_SHUTDOWN parameter uint16; debounceCount local uint16 =0; level_WarningEOP input uint16; level_ShutdownEOP input uint16; CD_SHUTDOWN_FUNCTION parameter uint8; CD_PRESSURE_HYSTERESIS parameter uint16; ENGINE_RUNNING parameter uint8; EXTREMELY_LOW parameter uint8; LOW parameter uint8; NORMAL parameter uint8; OORH parameter uint8; OORL parameter uint8; SHUTDOWN parameter uint8; NON_FUNCTIONAL parameter uint8; state_System input uint8; SYS_NORMAL parameter uint8; flt_EOP output uint16; CD_ECU_FUNCTION parameter uint8; ecu_EOP input uint8; state_CommECU input uint8; NO_COMMUNICATION parameter uint8; CD_EOP_FAULT_DETECTION_FUNCTION parameter uint8; CD_EOP_SWITCH_LOW parameter uint16

== matlab function MX_Gtwy_Control/System/AutoIdle/AutoIdleTime ==
  data: dlx_G55_AutoIdleTime input uint8; AutoIdleTime output uint16; CD_AUTOIDLE_IDLE_DELAY parameter uint16; ee_autoIdleTime data_store_memory double; CD_DELUXE_GEN5_5_FUNCTION parameter uint8; ee_DeluxeG5 data_store_memory double; NON_FUNCTIONAL parameter uint8; CD_DELUXE_GEN5_FUNCTION parameter uint8; ACTIVE parameter uint8
function AutoIdleTime = fcn(dlx_G55_AutoIdleTime, CD_AUTOIDLE_IDLE_DELAY, CD_DELUXE_GEN5_5_FUNCTION, NON_FUNCTIONAL, CD_DELUXE_GEN5_FUNCTION, ACTIVE)

aVarTruthTableCondition_1 = false;
aVarTruthTableCondition_2 = false;
aVarTruthTableCondition_3 = false;
aVarTruthTableCondition_4 = false;


% Gen5_5 Deluxe Display

aVarTruthTableCondition_1 = logical(CD_DELUXE_GEN5_5_FUNCTION ~= NON_FUNCTIONAL);

% Gen5 Deluxe Display

aVarTruthTableCondition_2 = logical(CD_DELUXE_GEN5_FUNCTION ~= NON_FUNCTIONAL && ee_DeluxeG5 == ACTIVE);

% Gen5_5 data valid

aVarTruthTableCondition_3 = logical(dlx_G55_AutoIdleTime >= 4  && dlx_G55_AutoIdleTime <=250);

% Gen5 data valid

aVarTruthTableCondition_4 = logical(ee_autoIdleTime >= 4  && ee_autoIdleTime <=250);

if (aVarTruthTableCondition_1 && aVarTruthTableCondition_3)
    GEN5_5();
elseif (aVarTruthTableCondition_2 && aVarTruthTableCondition_4)
    GEN5();
else % Default
    STANDARD();
end

function GEN5_5()

% Use can value
% (x10 for 100msec counts)
% Gen5_5 Display

AutoIdleTime = uint16(dlx_G55_AutoIdleTime) * 10;

function GEN5()

% Use eeprom value
% (x10 for 100msec counts)
% Gen5 Display

AutoIdleTime = uint16(ee_autoIdleTime) * 10;

function STANDARD()

% Use constant data
% Standard Display

AutoIdleTime = CD_AUTOIDLE_IDLE_DELAY;
